simulink model "AcasXuClosedLoop_all_2017b"  (Simulink 9.0)
settings: Solver=VariableStepAuto  StartTime=0.0  StopTime=20  RelTol=1e-3
libraries: neural x14040, simulink x2
(part 23 of 534)

== system AcasXuClosedLoop_all_2017b/networks/Networks_m_5/AcasXu_3_5/Process Output 1 ==
  a : Inport  PortDimensions=5
      mask Display: e = 0.06;c1 = [120 230 180]/255;c2 = ([120 230 180]+0)/(256+128);c3 = ([120 230 180]+128)/(256+128);patch([0 1 1 0],[0 0 1 1],c1);patch([0 1 1 1-e 1-e 0+e],[0 0 1 1-e 0+e 0+e],c2);patch([0 0 1 1-e 0+e 0+e],[0 1 1 1-e 1-e 0+e],c3);plot([0 1 1 0 0],[0 0 1 1 0]);
  y : Outport  PortDimensions=5
      mask Display: e = 0.06;c1 = [120 230 180]/255;c2 = ([120 230 180]+0)/(256+128);c3 = ([120 230 180]+128)/(256+128);patch([0 1 1 0],[0 0 1 1],c1);patch([0 1 1 1-e 1-e 0+e],[0 0 1 1-e 0+e 0+e],c2);patch([0 0 1 1-e 0+e 0+e],[0 1 1 1-e 1-e 0+e],c3);plot([0 1 1 0 0],[0 0 1 1 0]);
  wires:
  a/1 -> y/1

== system AcasXuClosedLoop_all_2017b/networks/Networks_m_5/AcasXu_4_5/Layer 1 ==
  "p{1}" : Inport  PortDimensions=5
      mask Display: e = 0.06;c1 = [120 230 180]/255;c2 = ([120 230 180]+0)/(256+128);c3 = ([120 230 180]+128)/(256+128);patch([0 1 1 0],[0 0 1 1],c1);patch([0 1 1 1-e 1-e 0+e],[0 0 1 1-e 0+e 0+e],c2);patch([0 0 1 1-e 0+e 0+e],[0 1 1 1-e 1-e 0+e],c3);plot([0 1 1 0 0],[0 0 1 1 0]);
  Delays 1 : Subsystem
      mask Display: e = 0.06;c1 = [120 150 230]/255;c2 = ([120 150 230]+0)/383;c3 = ([120 150 230]+128)/383;patch([0 1 1 0],[0 0 1 1],c1);patch([0 1 1 1-e 1-e 0+e],[0 0 1 1-e 0+e 0+e],c2);patch([0 0 1 1-e 0+e 0+e],[0 1 1 1-e 1-e 0+e],c3);plot([0 1 1 0 0],[0 0 1 1 0]);disp('0');
  "IW{1,1}" : Subsystem
      mask Display: e = 0.06;c1 = [120 150 230]/255;c2 = ([120 150 230]+0)/383;c3 = ([120 150 230]+128)/383;patch([0 1 1 0],[0 0 1 1],c1);patch([0 1 1 1-e 1-e 0+e],[0 0 1 1-e 0+e 0+e],c2);patch([0 0 1 1-e 0+e 0+e],[0 1 1 1-e 1-e 0+e],c3);plot([0 1 1 0 0],[0 0 1 1 0]);disp('W');
  "b{1}" : Constant  Value=[-0.55702700000000004987299462300143204629421234130859375;-0.317043000000000019245049998062313534319400787353515625;0.1381040000000000045332626541494391858577728271484375;0.1899780000000000079740658520677243359386920928955078125;0.18814900000000001067945731847430579364299774169921875;0.05523619999999999918838256007802556268870830535888671875;-0.06766999999999999404476369591066031716763973236083984375;-0.260805000000000009041656312547274865210056304931640625;-0.267183000000000003826272632068139500916004180908203125;-0.34851799999999999446487208842881955206394195556640625;-0.61407800000000001272582039746339432895183563232421875;0.2179210000000000035935698861067066900432109832763671875;-0.156318000000000012494894008341361768543720245361328125;-0.191004000000000007108980071279802359640598297119140625;-0.56346700000000005115197154736961238086223602294921875;0.198722000000000009745093620949774049222469329833984375;-0.64252600000000004154543375989305786788463592529296875;-0.057572900000000003239986057224086835049092769622802734375;0.05113020000000000064854788206503144465386867523193359375;-0.187771999999999994468424802107620052993297576904296875;-0.024837800000000000155697676973431953229010105133056640625;-0.02066489999999999993551824672977090813219547271728515625;0.25938600000000000544986278327996842563152313232421875;-0.1834780000000000022009061240169103257358074188232421875;-0.1499230000000000007087663789206999354064464569091796875;0.045113599999999996759125764356213039718568325042724609375;-0.3716880000000000183746351467561908066272735595703125;-0.0188616999999999986059595613596684415824711322784423828125;0.036677700000000000579714054538271739147603511810302734375;-0.552902000000000004575895218295045197010040283203125;0.18997900000000000897415475265006534755229949951171875;-0.3588970000000000215578666029614396393299102783203125;0.256363999999999980783371711368090473115444183349609375;0.138195000000000012274625760255730710923671722412109375;-0.47843400000000002592059900052845478057861328125;0.01338660000000000023512303215511565213091671466827392578125;-0.13313099999999999933919525574310682713985443115234375;-0.34949200000000002486189032424590550363063812255859375;-0.01373609999999999935205163836826613987796008586883544921875;-0.65224700000000002120259523508138954639434814453125;0.1802229999999999943138817570797982625663280487060546875;-0.408499000000000000998312543742940761148929595947265625;-0.42954199999999997938715523559949360787868499755859375;-0.02018129999999999923954163705275277607142925262451171875;0.07101649999999999629540781143077765591442584991455078125;0.06858029999999999681836726495021139271557331085205078125;-0.303010999999999974807707303625647909939289093017578125;-0.0773268000000000010896172852881136350333690643310546875;0.029496399999999999008704065772690228186547756195068359375;-0.2007400000000000017674750552032492123544216156005859375]
      mask Display: e = 0.06;c1 = [120 150 230]/255;c2 = ([120 150 230]+0)/383;c3 = ([120 150 230]+128)/383;patch([0 1 1 0],[0 0 1 1],c1);patch([0 1 1 1-e 1-e 0+e],[0 0 1 1-e 0+e 0+e],c2);patch([0 0 1 1-e 0+e 0+e],[0 1 1 1-e 1-e 0+e],c3);plot([0 1 1 0 0],[0 0 1 1 0]);disp('b');
  netsum : Sum  Inputs=2  SaturateOnIntegerOverflow=on  InputSameDT=on  OutDataTypeStr=Inherit: Same as first input
      mask Display: e = 0.06;c1 = [120 150 230]/255;c2 = ([120 150 230]+0)/383;c3 = ([120 150 230]+128)/383;patch([0 1 1 0],[0 0 1 1],c1);patch([0 1 1 1-e 1-e 0+e],[0 0 1 1-e 0+e 0+e],c2);patch([0 0 1 1-e 0+e 0+e],[0 1 1 1-e 1-e 0+e],c3);plot([0 1 1 0 0],[0 0 1 1 0]);disp('+');
  poslin : neural/Transfer Functions/poslin
      settings: SourceProductName=Deep Learning Toolbox  SourceProductBaseCode=NN
  "a{1}" : Outport  InitialOutput=[0;0;0;0;0;0;0;0;0;0;0;0;0;0;0;0;0;0;0;0;0;0;0;0;0;0;0;0;0;0;0;0;0;0;0;0;0;0;0;0;0;0;0;0;0;0;0;0;0;0]
      mask Display: e = 0.06;c1 = [120 230 180]/255;c2 = ([120 230 180]+0)/(256+128);c3 = ([120 230 180]+128)/(256+128);patch([0 1 1 0],[0 0 1 1],c1);patch([0 1 1 1-e 1-e 0+e],[0 0 1 1-e 0+e 0+e],c2);patch([0 0 1 1-e 0+e 0+e],[0 1 1 1-e 1-e 0+e],c3);plot([0 1 1 0 0],[0 0 1 1 0]);
  wires:
  poslin/1 -> "a{1}"/1
  netsum/1 -> poslin/1
  "b{1}"/1 -> netsum/2
  "IW{1,1}"/1("iz{1,1}") -> netsum/1
  Delays 1/1("pd{1,1}") -> "IW{1,1}"/1("pd{1,1}")
  "p{1}"/1 -> Delays 1/1("p{1}")

== system AcasXuClosedLoop_all_2017b/networks/Networks_m_5/AcasXu_4_5/Layer 2 ==
  "a{1} " : Inport  PortDimensions=50
      mask Display: e = 0.06;c1 = [120 230 180]/255;c2 = ([120 230 180]+0)/(256+128);c3 = ([120 230 180]+128)/(256+128);patch([0 1 1 0],[0 0 1 1],c1);patch([0 1 1 1-e 1-e 0+e],[0 0 1 1-e 0+e 0+e],c2);patch([0 0 1 1-e 0+e 0+e],[0 1 1 1-e 1-e 0+e],c3);plot([0 1 1 0 0],[0 0 1 1 0]);
  Delays 1 : Subsystem
      mask Display: e = 0.06;c1 = [120 150 230]/255;c2 = ([120 150 230]+0)/383;c3 = ([120 150 230]+128)/383;patch([0 1 1 0],[0 0 1 1],c1);patch([0 1 1 1-e 1-e 0+e],[0 0 1 1-e 0+e 0+e],c2);patch([0 0 1 1-e 0+e 0+e],[0 1 1 1-e 1-e 0+e],c3);plot([0 1 1 0 0],[0 0 1 1 0]);disp('0');
  "LW{2,1}" : Subsystem
      mask Display: e = 0.06;c1 = [120 150 230]/255;c2 = ([120 150 230]+0)/383;c3 = ([120 150 230]+128)/383;patch([0 1 1 0],[0 0 1 1],c1);patch([0 1 1 1-e 1-e 0+e],[0 0 1 1-e 0+e 0+e],c2);patch([0 0 1 1-e 0+e 0+e],[0 1 1 1-e 1-e 0+e],c3);plot([0 1 1 0 0],[0 0 1 1 0]);disp('W');
  "b{2}" : Constant  Value=[-0.72558800000000001073630073733511380851268768310546875;-0.0030770799999999998326771777357180326362140476703643798828125;-0.0214728999999999996262101120692022959701716899871826171875;-1.022629999999999927950966593925841152667999267578125;0.013211699999999999832756003570466418750584125518798828125;0.254539000000000015244694395732949487864971160888671875;-0.0161836999999999987809307100405931123532354831695556640625;1.004890000000000060964566728216595947742462158203125;0.366732999999999975671016727574169635772705078125;0.5765860000000000429309920946252532303333282470703125;-1.1223099999999999187849653026205487549304962158203125;-0.1011060000000000014264145420384011231362819671630859375;0.2349920000000000064321881154683069325983524322509765625;0.4978920000000000012363443602225743234157562255859375;0.380083000000000004181543999948189593851566314697265625;-0.1062639999999999973478992387754260562360286712646484375;-0.00875836999999999969712671799015879514627158641815185546875;-0.2173940000000000039026559761623502708971500396728515625;-0.03386909999999999920650139983990811742842197418212890625;0.1742410000000000069864114493611850775778293609619140625;0.9405099999999999571542730336659587919712066650390625;-0.446786999999999989707788472514948807656764984130859375;-0.432205000000000005844214001626824028789997100830078125;-0.67214099999999998846789139861357398331165313720703125;-0.847107999999999972118303048773668706417083740234375;-1.2810200000000000475353090223507024347782135009765625;-0.0116062000000000005439648731453416985459625720977783203125;-0.0695950999999999930789584823287441395223140716552734375;0.76355300000000003723954478118685074150562286376953125;-0.0096115499999999999547473095162786194123327732086181640625;-0.455886999999999986687981845534523017704486846923828125;-0.88311499999999998333777284642565064132213592529296875;-0.1558090000000000030500046932502300478518009185791015625;-0.2641649999999999831601371624856255948543548583984375;1.152320000000000010942358130705542862415313720703125;-0.00141853000000000009465928041407778437132947146892547607421875;-0.1892749999999999988009591334048309363424777984619140625;0.00461054999999999971793673836373272933997213840484619140625;-0.033554899999999998616573293475084938108921051025390625;-0.0259916000000000001202149491064119501970708370208740234375;-0.62039299999999997226041159592568874359130859375;-0.5875559999999999671871364625985734164714813232421875;0.2564100000000000267874611381557770073413848876953125;0.323012000000000021326940213839407078921794891357421875;-0.339326999999999989743315609302953816950321197509765625;0.085024299999999997101696180834551341831684112548828125;-0.09253550000000000663735733041903586126863956451416015625;0.110709000000000001850963826655060984194278717041015625;-0.006517229999999999724702437475798433297313749790191650390625;0.7578899999999999526067995248013176023960113525390625]
      mask Display: e = 0.06;c1 = [120 150 230]/255;c2 = ([120 150 230]+0)/383;c3 = ([120 150 230]+128)/383;patch([0 1 1 0],[0 0 1 1],c1);patch([0 1 1 1-e 1-e 0+e],[0 0 1 1-e 0+e 0+e],c2);patch([0 0 1 1-e 0+e 0+e],[0 1 1 1-e 1-e 0+e],c3);plot([0 1 1 0 0],[0 0 1 1 0]);disp('b');
  netsum : Sum  Inputs=2  SaturateOnIntegerOverflow=on  InputSameDT=on  OutDataTypeStr=Inherit: Same as first input
      mask Display: e = 0.06;c1 = [120 150 230]/255;c2 = ([120 150 230]+0)/383;c3 = ([120 150 230]+128)/383;patch([0 1 1 0],[0 0 1 1],c1);patch([0 1 1 1-e 1-e 0+e],[0 0 1 1-e 0+e 0+e],c2);patch([0 0 1 1-e 0+e 0+e],[0 1 1 1-e 1-e 0+e],c3);plot([0 1 1 0 0],[0 0 1 1 0]);disp('+');
  poslin : neural/Transfer Functions/poslin
      settings: SourceProductName=Deep Learning Toolbox  SourceProductBaseCode=NN
  "a{2}" : Outport  InitialOutput=[0;0;0;0;0;0;0;0;0;0;0;0;0;0;0;0;0;0;0;0;0;0;0;0;0;0;0;0;0;0;0;0;0;0;0;0;0;0;0;0;0;0;0;0;0;0;0;0;0;0]
      mask Display: e = 0.06;c1 = [120 230 180]/255;c2 = ([120 230 180]+0)/(256+128);c3 = ([120 230 180]+128)/(256+128);patch([0 1 1 0],[0 0 1 1],c1);patch([0 1 1 1-e 1-e 0+e],[0 0 1 1-e 0+e 0+e],c2);patch([0 0 1 1-e 0+e 0+e],[0 1 1 1-e 1-e 0+e],c3);plot([0 1 1 0 0],[0 0 1 1 0]);
  wires:
  poslin/1 -> "a{2}"/1
  netsum/1 -> poslin/1
  "b{2}"/1 -> netsum/2
  "LW{2,1}"/1("lz{2,1}") -> netsum/1
  Delays 1/1("pd{2,1}") -> "LW{2,1}"/1("ad{2,1}")
  "a{1} "/1 -> Delays 1/1("p{2}")

== system AcasXuClosedLoop_all_2017b/networks/Networks_m_5/AcasXu_4_5/Layer 3 ==
  "a{2} " : Inport  PortDimensions=50
      mask Display: e = 0.06;c1 = [120 230 180]/255;c2 = ([120 230 180]+0)/(256+128);c3 = ([120 230 180]+128)/(256+128);patch([0 1 1 0],[0 0 1 1],c1);patch([0 1 1 1-e 1-e 0+e],[0 0 1 1-e 0+e 0+e],c2);patch([0 0 1 1-e 0+e 0+e],[0 1 1 1-e 1-e 0+e],c3);plot([0 1 1 0 0],[0 0 1 1 0]);
  Delays 1 : Subsystem
      mask Display: e = 0.06;c1 = [120 150 230]/255;c2 = ([120 150 230]+0)/383;c3 = ([120 150 230]+128)/383;patch([0 1 1 0],[0 0 1 1],c1);patch([0 1 1 1-e 1-e 0+e],[0 0 1 1-e 0+e 0+e],c2);patch([0 0 1 1-e 0+e 0+e],[0 1 1 1-e 1-e 0+e],c3);plot([0 1 1 0 0],[0 0 1 1 0]);disp('0');
  "LW{3,2}" : Subsystem
      mask Display: e = 0.06;c1 = [120 150 230]/255;c2 = ([120 150 230]+0)/383;c3 = ([120 150 230]+128)/383;patch([0 1 1 0],[0 0 1 1],c1);patch([0 1 1 1-e 1-e 0+e],[0 0 1 1-e 0+e 0+e],c2);patch([0 0 1 1-e 0+e 0+e],[0 1 1 1-e 1-e 0+e],c3);plot([0 1 1 0 0],[0 0 1 1 0]);disp('W');
  "b{3}" : Constant  Value=[1.2183299999999999130295691429637372493743896484375;0.3758529999999999926529881122405640780925750732421875;-0.49951800000000001755751100063207559287548065185546875;0.2297079999999999955218044078719685785472393035888671875;-0.2305609999999999881747925201125326566398143768310546875;0.6040140000000000508606490257079713046550750732421875;-0.1264039999999999885904600205321912653744220733642578125;-1.15477000000000007418066161335445940494537353515625;0.9251979999999999648707671440206468105316162109375;0.391276000000000012679635119638987816870212554931640625;-0.392373000000000027309710048939450643956661224365234375;-0.0485302999999999984392928809029399417340755462646484375;0.661183999999999993946175891323946416378021240234375;-0.258817000000000019266366280135116539895534515380859375;-0.2621430000000000148929757415316998958587646484375;-0.421345000000000025064395003937534056603908538818359375;-2.23233000000000014750867194379679858684539794921875;-0.402540999999999982161824618742684833705425262451171875;-1.2703599999999999337063627535826526582241058349609375;0.62634800000000001585220843480783514678478240966796875;-1.4001799999999999801758576722932048141956329345703125;-0.9811549999999999993605115378159098327159881591796875;-1.5831999999999999406696815640316344797611236572265625;0.06447740000000000415436574030536576174199581146240234375;0.269380000000000008331113576787174679338932037353515625;-0.363032999999999994589217067186837084591388702392578125;-0.00779845000000000017348344982792696100659668445587158203125;-0.55507899999999998907895815136726014316082000732421875;0.85643199999999997107380522720632143318653106689453125;0.2440710000000000101660901918876334093511104583740234375;-0.340949000000000002064126647383091039955615997314453125;1.81261999999999989796606314484961330890655517578125;0.86188100000000000822097945274435915052890777587890625;0.70267800000000002480504690538509748876094818115234375;-2.120080000000000186588522410602308809757232666015625;-0.47471900000000000208189021577709354460239410400390625;0.73649100000000000676436684443615376949310302734375;-0.19158500000000000529354338141274638473987579345703125;-0.99656299999999997663024942085030488669872283935546875;-1.2982299999999999950972551232553087174892425537109375;-0.3749730000000000007531752999057061970233917236328125;0.355833000000000010398792937849066220223903656005859375;0.46674300000000001897859647215227596461772918701171875;0.195896999999999987807086654356680810451507568359375;-0.2098629999999999939941375259877531789243221282958984375;1.44169999999999998152588887023739516735076904296875;-1.926909999999999900666125540737994015216827392578125;-1.3318600000000000438404867963981814682483673095703125;-0.60810799999999998188826566547504626214504241943359375;-0.055248899999999996623945008877853979356586933135986328125]
      mask Display: e = 0.06;c1 = [120 150 230]/255;c2 = ([120 150 230]+0)/383;c3 = ([120 150 230]+128)/383;patch([0 1 1 0],[0 0 1 1],c1);patch([0 1 1 1-e 1-e 0+e],[0 0 1 1-e 0+e 0+e],c2);patch([0 0 1 1-e 0+e 0+e],[0 1 1 1-e 1-e 0+e],c3);plot([0 1 1 0 0],[0 0 1 1 0]);disp('b');
  netsum : Sum  Inputs=2  SaturateOnIntegerOverflow=on  InputSameDT=on  OutDataTypeStr=Inherit: Same as first input
      mask Display: e = 0.06;c1 = [120 150 230]/255;c2 = ([120 150 230]+0)/383;c3 = ([120 150 230]+128)/383;patch([0 1 1 0],[0 0 1 1],c1);patch([0 1 1 1-e 1-e 0+e],[0 0 1 1-e 0+e 0+e],c2);patch([0 0 1 1-e 0+e 0+e],[0 1 1 1-e 1-e 0+e],c3);plot([0 1 1 0 0],[0 0 1 1 0]);disp('+');
  poslin : neural/Transfer Functions/poslin
      settings: SourceProductName=Deep Learning Toolbox  SourceProductBaseCode=NN
  "a{3}" : Outport  InitialOutput=[0;0;0;0;0;0;0;0;0;0;0;0;0;0;0;0;0;0;0;0;0;0;0;0;0;0;0;0;0;0;0;0;0;0;0;0;0;0;0;0;0;0;0;0;0;0;0;0;0;0]
      mask Display: e = 0.06;c1 = [120 230 180]/255;c2 = ([120 230 180]+0)/(256+128);c3 = ([120 230 180]+128)/(256+128);patch([0 1 1 0],[0 0 1 1],c1);patch([0 1 1 1-e 1-e 0+e],[0 0 1 1-e 0+e 0+e],c2);patch([0 0 1 1-e 0+e 0+e],[0 1 1 1-e 1-e 0+e],c3);plot([0 1 1 0 0],[0 0 1 1 0]);
  wires:
  poslin/1 -> "a{3}"/1
  netsum/1 -> poslin/1
  "b{3}"/1 -> netsum/2
  "LW{3,2}"/1("lz{3,2}") -> netsum/1
  Delays 1/1("pd{3,2}") -> "LW{3,2}"/1("ad{3,2}")
  "a{2} "/1 -> Delays 1/1("p{3}")

== system AcasXuClosedLoop_all_2017b/networks/Networks_m_5/AcasXu_4_5/Layer 4 ==
  "a{3} " : Inport  PortDimensions=50
      mask Display: e = 0.06;c1 = [120 230 180]/255;c2 = ([120 230 180]+0)/(256+128);c3 = ([120 230 180]+128)/(256+128);patch([0 1 1 0],[0 0 1 1],c1);patch([0 1 1 1-e 1-e 0+e],[0 0 1 1-e 0+e 0+e],c2);patch([0 0 1 1-e 0+e 0+e],[0 1 1 1-e 1-e 0+e],c3);plot([0 1 1 0 0],[0 0 1 1 0]);
  Delays 1 : Subsystem
      mask Display: e = 0.06;c1 = [120 150 230]/255;c2 = ([120 150 230]+0)/383;c3 = ([120 150 230]+128)/383;patch([0 1 1 0],[0 0 1 1],c1);patch([0 1 1 1-e 1-e 0+e],[0 0 1 1-e 0+e 0+e],c2);patch([0 0 1 1-e 0+e 0+e],[0 1 1 1-e 1-e 0+e],c3);plot([0 1 1 0 0],[0 0 1 1 0]);disp('0');
  "LW{4,3}" : Subsystem
      mask Display: e = 0.06;c1 = [120 150 230]/255;c2 = ([120 150 230]+0)/383;c3 = ([120 150 230]+128)/383;patch([0 1 1 0],[0 0 1 1],c1);patch([0 1 1 1-e 1-e 0+e],[0 0 1 1-e 0+e 0+e],c2);patch([0 0 1 1-e 0+e 0+e],[0 1 1 1-e 1-e 0+e],c3);plot([0 1 1 0 0],[0 0 1 1 0]);disp('W');
  "b{4}" : Constant  Value=[-1.13694000000000006167510946397669613361358642578125;-1.44306000000000000937916411203332245349884033203125;-0.460143000000000024218849148383014835417270660400390625;-0.0907355999999999995875299418912618421018123626708984375;0.2415279999999999926973970332255703397095203399658203125;-0.192749000000000003662847802843316458165645599365234375;-0.6306410000000000071196382123162038624286651611328125;0.83967000000000002746247673712787218391895294189453125;-1.3998500000000000387245790989254601299762725830078125;0.0822589999999999987867482786896289326250553131103515625;0.64448600000000000331823457599966786801815032958984375;0.346046000000000020246915255484054796397686004638671875;-1.373180000000000067217342802905477583408355712890625;1.917710000000000025721647034515626728534698486328125;1.3785000000000000586197757002082653343677520751953125;1.09443000000000001392663762089796364307403564453125;1.95282000000000000028421709430404007434844970703125;0.1447380000000000055404569820893811993300914764404296875;-2.013619999999999965467623042059130966663360595703125;0.333592999999999972882136489715776406228542327880859375;-0.01296309999999999997000177387462827027775347232818603515625;1.175580000000000069348971010185778141021728515625;0.07237180000000000001936228954946273006498813629150390625;-1.059649999999999980815346134477294981479644775390625;-0.9808179999999999676418838134850375354290008544921875;-3.806229999999999780158077555825002491474151611328125;-2.06843999999999983430143402074463665485382080078125;-3.407760000000000122355459097889252007007598876953125;-1.9770700000000001050892706189188174903392791748046875;0.5420409999999999950404117043945007026195526123046875;-2.08537000000000016797230273368768393993377685546875;-2.5549400000000002108890839735977351665496826171875;-1.272710000000000007958078640513122081756591796875;1.8852100000000000523669996255193836987018585205078125;0.417991999999999974679809611188829876482486724853515625;-1.0045699999999999629807234668987803161144256591796875;4.056779999999999830606611794792115688323974609375;-1.4069599999999999884181534071103669703006744384765625;-1.2732200000000000184030568561865948140621185302734375;0.00521946000000000041862957544935852638445794582366943359375;-0.06468649999999999400390748860445455648005008697509765625;-0.11416099999999999858868449109650100581347942352294921875;-0.78644800000000003592504072003066539764404296875;1.2535000000000000586197757002082653343677520751953125;-1.5074199999999999821653773324214853346347808837890625;-0.2420319999999999971418418454049970023334026336669921875;1.3262300000000000199662508748588152229785919189453125;0.19241099999999999869970679355901665985584259033203125;-0.0200379000000000008496758852061248035170137882232666015625;-1.84077000000000001733724275254644453525543212890625]
      mask Display: e = 0.06;c1 = [120 150 230]/255;c2 = ([120 150 230]+0)/383;c3 = ([120 150 230]+128)/383;patch([0 1 1 0],[0 0 1 1],c1);patch([0 1 1 1-e 1-e 0+e],[0 0 1 1-e 0+e 0+e],c2);patch([0 0 1 1-e 0+e 0+e],[0 1 1 1-e 1-e 0+e],c3);plot([0 1 1 0 0],[0 0 1 1 0]);disp('b');
  netsum : Sum  Inputs=2  SaturateOnIntegerOverflow=on  InputSameDT=on  OutDataTypeStr=Inherit: Same as first input
      mask Display: e = 0.06;c1 = [120 150 230]/255;c2 = ([120 150 230]+0)/383;c3 = ([120 150 230]+128)/383;patch([0 1 1 0],[0 0 1 1],c1);patch([0 1 1 1-e 1-e 0+e],[0 0 1 1-e 0+e 0+e],c2);patch([0 0 1 1-e 0+e 0+e],[0 1 1 1-e 1-e 0+e],c3);plot([0 1 1 0 0],[0 0 1 1 0]);disp('+');
  poslin : neural/Transfer Functions/poslin
      settings: SourceProductName=Deep Learning Toolbox  SourceProductBaseCode=NN
  "a{4}" : Outport  InitialOutput=[0;0;0;0;0;0;0;0;0;0;0;0;0;0;0;0;0;0;0;0;0;0;0;0;0;0;0;0;0;0;0;0;0;0;0;0;0;0;0;0;0;0;0;0;0;0;0;0;0;0]
      mask Display: e = 0.06;c1 = [120 230 180]/255;c2 = ([120 230 180]+0)/(256+128);c3 = ([120 230 180]+128)/(256+128);patch([0 1 1 0],[0 0 1 1],c1);patch([0 1 1 1-e 1-e 0+e],[0 0 1 1-e 0+e 0+e],c2);patch([0 0 1 1-e 0+e 0+e],[0 1 1 1-e 1-e 0+e],c3);plot([0 1 1 0 0],[0 0 1 1 0]);
  wires:
  poslin/1 -> "a{4}"/1
  netsum/1 -> poslin/1
  "b{4}"/1 -> netsum/2
  "LW{4,3}"/1("lz{4,3}") -> netsum/1
  Delays 1/1("pd{4,3}") -> "LW{4,3}"/1("ad{4,3}")
  "a{3} "/1 -> Delays 1/1("p{4}")

== system AcasXuClosedLoop_all_2017b/networks/Networks_m_5/AcasXu_4_5/Layer 5 ==
  "a{4} " : Inport  PortDimensions=50
      mask Display: e = 0.06;c1 = [120 230 180]/255;c2 = ([120 230 180]+0)/(256+128);c3 = ([120 230 180]+128)/(256+128);patch([0 1 1 0],[0 0 1 1],c1);patch([0 1 1 1-e 1-e 0+e],[0 0 1 1-e 0+e 0+e],c2);patch([0 0 1 1-e 0+e 0+e],[0 1 1 1-e 1-e 0+e],c3);plot([0 1 1 0 0],[0 0 1 1 0]);
  Delays 1 : Subsystem
      mask Display: e = 0.06;c1 = [120 150 230]/255;c2 = ([120 150 230]+0)/383;c3 = ([120 150 230]+128)/383;patch([0 1 1 0],[0 0 1 1],c1);patch([0 1 1 1-e 1-e 0+e],[0 0 1 1-e 0+e 0+e],c2);patch([0 0 1 1-e 0+e 0+e],[0 1 1 1-e 1-e 0+e],c3);plot([0 1 1 0 0],[0 0 1 1 0]);disp('0');
  "LW{5,4}" : Subsystem
      mask Display: e = 0.06;c1 = [120 150 230]/255;c2 = ([120 150 230]+0)/383;c3 = ([120 150 230]+128)/383;patch([0 1 1 0],[0 0 1 1],c1);patch([0 1 1 1-e 1-e 0+e],[0 0 1 1-e 0+e 0+e],c2);patch([0 0 1 1-e 0+e 0+e],[0 1 1 1-e 1-e 0+e],c3);plot([0 1 1 0 0],[0 0 1 1 0]);disp('W');
  "b{5}" : Constant  Value=[-0.005314890000000000065683014582873511244542896747589111328125;1.0904199999999999448618837050162255764007568359375;2.140670000000000072759576141834259033203125;1.008620000000000072049033406074158847332000732421875;2.096159999999999801190142534323967993259429931640625;2.171110000000000095354835139005444943904876708984375;0.60804800000000003290523409305023960769176483154296875;-0.4575299999999999922550841802149079740047454833984375;2.1910199999999999675992512493394315242767333984375;-1.6480799999999999894129132371745072305202484130859375;-0.499765999999999988023802188763511367142200469970703125;0.85345199999999998841104797975276596844196319580078125;2.008459999999999912034809312899596989154815673828125;-0.0260502999999999984570564492969424463808536529541015625;2.183240000000000069491079557337798178195953369140625;0.2656549999999999744915157862124033272266387939453125;0.9520910000000000206199501917581073939800262451171875;0.5391049999999999453592636200482957065105438232421875;3.45282000000000000028421709430404007434844970703125;0.6617189999999999461266497746692039072513580322265625;-0.81202600000000002555822220529080368578433990478515625;-0.66264599999999995727506529874517582356929779052734375;-0.038156900000000000539035482916006003506481647491455078125;3.081989999999999785273985253297723829746246337890625;0.259077000000000001733724275254644453525543212890625;0.5673280000000000544702061233692802488803863525390625;0.11513299999999999922994931011999142356216907501220703125;4.59682000000000012818190953112207353115081787109375;0;-1.0043699999999999850075482754618860781192779541015625;-2.511760000000000214726014746702276170253753662109375;0.59331999999999995853983136839815415441989898681640625;1.174630000000000062954086388344876468181610107421875;0.49534000000000000252242671194835565984249114990234375;-1.8436500000000000110134124042815528810024261474609375;2.802049999999999929656269159750081598758697509765625;-0.397106000000000014527046232615248300135135650634765625;-0.168563999999999991619148431709618307650089263916015625;1.7117500000000001048050535246147774159908294677734375;1.1961500000000000465405491922865621745586395263671875;1.292710000000000025721647034515626728534698486328125;2.4907599999999998630073605454526841640472412109375;-0.335679999999999978399500832892954349517822265625;1.2010099999999999109689952092594467103481292724609375;0.98422500000000001652011860642232932150363922119140625;0.92655200000000004223466021358035504817962646484375;3.378190000000000026147972675971686840057373046875;1.092610000000000081143980423803441226482391357421875;-1.2016899999999999248956328301574103534221649169921875;0.1211689999999999989288568258416489697992801666259765625]
      mask Display: e = 0.06;c1 = [120 150 230]/255;c2 = ([120 150 230]+0)/383;c3 = ([120 150 230]+128)/383;patch([0 1 1 0],[0 0 1 1],c1);patch([0 1 1 1-e 1-e 0+e],[0 0 1 1-e 0+e 0+e],c2);patch([0 0 1 1-e 0+e 0+e],[0 1 1 1-e 1-e 0+e],c3);plot([0 1 1 0 0],[0 0 1 1 0]);disp('b');
  netsum : Sum  Inputs=2  SaturateOnIntegerOverflow=on  InputSameDT=on  OutDataTypeStr=Inherit: Same as first input
      mask Display: e = 0.06;c1 = [120 150 230]/255;c2 = ([120 150 230]+0)/383;c3 = ([120 150 230]+128)/383;patch([0 1 1 0],[0 0 1 1],c1);patch([0 1 1 1-e 1-e 0+e],[0 0 1 1-e 0+e 0+e],c2);patch([0 0 1 1-e 0+e 0+e],[0 1 1 1-e 1-e 0+e],c3);plot([0 1 1 0 0],[0 0 1 1 0]);disp('+');
  poslin : neural/Transfer Functions/poslin
      settings: SourceProductName=Deep Learning Toolbox  SourceProductBaseCode=NN
  "a{5}" : Outport  InitialOutput=[0;0;0;0;0;0;0;0;0;0;0;0;0;0;0;0;0;0;0;0;0;0;0;0;0;0;0;0;0;0;0;0;0;0;0;0;0;0;0;0;0;0;0;0;0;0;0;0;0;0]
      mask Display: e = 0.06;c1 = [120 230 180]/255;c2 = ([120 230 180]+0)/(256+128);c3 = ([120 230 180]+128)/(256+128);patch([0 1 1 0],[0 0 1 1],c1);patch([0 1 1 1-e 1-e 0+e],[0 0 1 1-e 0+e 0+e],c2);patch([0 0 1 1-e 0+e 0+e],[0 1 1 1-e 1-e 0+e],c3);plot([0 1 1 0 0],[0 0 1 1 0]);
  wires:
  poslin/1 -> "a{5}"/1
  netsum/1 -> poslin/1
  "b{5}"/1 -> netsum/2
  "LW{5,4}"/1("lz{5,4}") -> netsum/1
  Delays 1/1("pd{5,4}") -> "LW{5,4}"/1("ad{5,4}")
  "a{4} "/1 -> Delays 1/1("p{5}")

== system AcasXuClosedLoop_all_2017b/networks/Networks_m_5/AcasXu_4_5/Layer 6 ==
  "a{5} " : Inport  PortDimensions=50
      mask Display: e = 0.06;c1 = [120 230 180]/255;c2 = ([120 230 180]+0)/(256+128);c3 = ([120 230 180]+128)/(256+128);patch([0 1 1 0],[0 0 1 1],c1);patch([0 1 1 1-e 1-e 0+e],[0 0 1 1-e 0+e 0+e],c2);patch([0 0 1 1-e 0+e 0+e],[0 1 1 1-e 1-e 0+e],c3);plot([0 1 1 0 0],[0 0 1 1 0]);
  Delays 1 : Subsystem
      mask Display: e = 0.06;c1 = [120 150 230]/255;c2 = ([120 150 230]+0)/383;c3 = ([120 150 230]+128)/383;patch([0 1 1 0],[0 0 1 1],c1);patch([0 1 1 1-e 1-e 0+e],[0 0 1 1-e 0+e 0+e],c2);patch([0 0 1 1-e 0+e 0+e],[0 1 1 1-e 1-e 0+e],c3);plot([0 1 1 0 0],[0 0 1 1 0]);disp('0');
  "LW{6,5}" : Subsystem
      mask Display: e = 0.06;c1 = [120 150 230]/255;c2 = ([120 150 230]+0)/383;c3 = ([120 150 230]+128)/383;patch([0 1 1 0],[0 0 1 1],c1);patch([0 1 1 1-e 1-e 0+e],[0 0 1 1-e 0+e 0+e],c2);patch([0 0 1 1-e 0+e 0+e],[0 1 1 1-e 1-e 0+e],c3);plot([0 1 1 0 0],[0 0 1 1 0]);disp('W');
  "b{6}" : Constant  Value=[0.2259790000000000131930022462256602011620998382568359375;0.64113699999999995693400478558032773435115814208984375;-1.122030000000000082849282989627681672573089599609375;-0.258402999999999993807620057850726880133152008056640625;0.279403999999999985703880156506784260272979736328125;-3.158129999999999881765688769519329071044921875;3.81989000000000000767386154620908200740814208984375;-0.83881700000000003480948862488730810582637786865234375;0.33712900000000001199396137963049113750457763671875;1.481430000000000024584778657299466431140899658203125;-0.387311000000000016374457345591508783400058746337890625;-4.85249000000000041410430640098638832569122314453125;-1.463179999999999925108795650885440409183502197265625;-0.62209700000000001107736125049996189773082733154296875;-5.7391600000000000392219590139575302600860595703125;-0.53950500000000001232791646543773822486400604248046875;0.355816999999999994397370528531610034406185150146484375;-3.2089400000000001256239556823857128620147705078125;-0.66979200000000005399414249041001312434673309326171875;-0.053271800000000001096278623435864574275910854339599609375;-1.0725899999999999323563315556384623050689697265625;0.2332220000000000126316734849751810543239116668701171875;0.54013299999999997424993125605396926403045654296875;0.034703799999999999925659466271099518053233623504638671875;-1.8084800000000000874678107720683328807353973388671875;-1.0016700000000000603250782660325057804584503173828125;-1.9715800000000001102051783163915388286113739013671875;1.993749999999999911182158029987476766109466552734375;0.66732599999999997475441659844364039599895477294921875;-2.41988000000000003097966327914036810398101806640625;-0.262247999999999981124432224532938562333583831787109375;-2.322389999999999954383156364201568067073822021484375;-6.11990999999999996106225808034650981426239013671875;-2.560119999999999951256768326857127249240875244140625;0.2439320000000000099316110890868003480136394500732421875;-0.2257039999999999879687351267421036027371883392333984375;-0.1239760000000000028652635819526039995253086090087890625;1.8548400000000000442668124378542415797710418701171875;-0.4922050000000000036237679523765109479427337646484375;-0.57257499999999994511057366253226064145565032958984375;-0.53078599999999998004795997985638678073883056640625;-0.59304900000000004833822231375961564481258392333984375;0.0029524999999999998294419878419603264774195849895477294921875;-3.167949999999999821653773324214853346347808837890625;-0.80684900000000003839062401311821304261684417724609375;-3.264060000000000183462134373257867991924285888671875;-0.874765000000000014779288903810083866119384765625;-2.214059999999999917008608463220298290252685546875;0.2967400000000000037658764995285309851169586181640625;-5.6659699999999997288568920339457690715789794921875]
      mask Display: e = 0.06;c1 = [120 150 230]/255;c2 = ([120 150 230]+0)/383;c3 = ([120 150 230]+128)/383;patch([0 1 1 0],[0 0 1 1],c1);patch([0 1 1 1-e 1-e 0+e],[0 0 1 1-e 0+e 0+e],c2);patch([0 0 1 1-e 0+e 0+e],[0 1 1 1-e 1-e 0+e],c3);plot([0 1 1 0 0],[0 0 1 1 0]);disp('b');
  netsum : Sum  Inputs=2  SaturateOnIntegerOverflow=on  InputSameDT=on  OutDataTypeStr=Inherit: Same as first input
      mask Display: e = 0.06;c1 = [120 150 230]/255;c2 = ([120 150 230]+0)/383;c3 = ([120 150 230]+128)/383;patch([0 1 1 0],[0 0 1 1],c1);patch([0 1 1 1-e 1-e 0+e],[0 0 1 1-e 0+e 0+e],c2);patch([0 0 1 1-e 0+e 0+e],[0 1 1 1-e 1-e 0+e],c3);plot([0 1 1 0 0],[0 0 1 1 0]);disp('+');
  poslin : neural/Transfer Functions/poslin
      settings: SourceProductName=Deep Learning Toolbox  SourceProductBaseCode=NN
  "a{6}" : Outport  InitialOutput=[0;0;0;0;0;0;0;0;0;0;0;0;0;0;0;0;0;0;0;0;0;0;0;0;0;0;0;0;0;0;0;0;0;0;0;0;0;0;0;0;0;0;0;0;0;0;0;0;0;0]
      mask Display: e = 0.06;c1 = [120 230 180]/255;c2 = ([120 230 180]+0)/(256+128);c3 = ([120 230 180]+128)/(256+128);patch([0 1 1 0],[0 0 1 1],c1);patch([0 1 1 1-e 1-e 0+e],[0 0 1 1-e 0+e 0+e],c2);patch([0 0 1 1-e 0+e 0+e],[0 1 1 1-e 1-e 0+e],c3);plot([0 1 1 0 0],[0 0 1 1 0]);
  wires:
  poslin/1 -> "a{6}"/1
  netsum/1 -> poslin/1
  "b{6}"/1 -> netsum/2
  "LW{6,5}"/1("lz{6,5}") -> netsum/1
  Delays 1/1("pd{6,5}") -> "LW{6,5}"/1("ad{6,5}")
  "a{5} "/1 -> Delays 1/1("p{6}")

== system AcasXuClosedLoop_all_2017b/networks/Networks_m_5/AcasXu_4_5/Layer 7 ==
  "a{6} " : Inport  PortDimensions=50
      mask Display: e = 0.06;c1 = [120 230 180]/255;c2 = ([120 230 180]+0)/(256+128);c3 = ([120 230 180]+128)/(256+128);patch([0 1 1 0],[0 0 1 1],c1);patch([0 1 1 1-e 1-e 0+e],[0 0 1 1-e 0+e 0+e],c2);patch([0 0 1 1-e 0+e 0+e],[0 1 1 1-e 1-e 0+e],c3);plot([0 1 1 0 0],[0 0 1 1 0]);
  Delays 1 : Subsystem
      mask Display: e = 0.06;c1 = [120 150 230]/255;c2 = ([120 150 230]+0)/383;c3 = ([120 150 230]+128)/383;patch([0 1 1 0],[0 0 1 1],c1);patch([0 1 1 1-e 1-e 0+e],[0 0 1 1-e 0+e 0+e],c2);patch([0 0 1 1-e 0+e 0+e],[0 1 1 1-e 1-e 0+e],c3);plot([0 1 1 0 0],[0 0 1 1 0]);disp('0');
  "LW{7,6}" : Subsystem
      mask Display: e = 0.06;c1 = [120 150 230]/255;c2 = ([120 150 230]+0)/383;c3 = ([120 150 230]+128)/383;patch([0 1 1 0],[0 0 1 1],c1);patch([0 1 1 1-e 1-e 0+e],[0 0 1 1-e 0+e 0+e],c2);patch([0 0 1 1-e 0+e 0+e],[0 1 1 1-e 1-e 0+e],c3);plot([0 1 1 0 0],[0 0 1 1 0]);disp('W');
  "b{7}" : Constant  Value=[-0.0206514999999999997182253963501352700404822826385498046875;-0.0190315999999999992120525149630339001305401325225830078125;0.01813789999999999846824749738516402430832386016845703125;-0.01843229999999999868531830315987463109195232391357421875;0.018363999999999998380406651676821638830006122589111328125]
      mask Display: e = 0.06;c1 = [120 150 230]/255;c2 = ([120 150 230]+0)/383;c3 = ([120 150 230]+128)/383;patch([0 1 1 0],[0 0 1 1],c1);patch([0 1 1 1-e 1-e 0+e],[0 0 1 1-e 0+e 0+e],c2);patch([0 0 1 1-e 0+e 0+e],[0 1 1 1-e 1-e 0+e],c3);plot([0 1 1 0 0],[0 0 1 1 0]);disp('b');
  netsum : Sum  Inputs=2  SaturateOnIntegerOverflow=on  InputSameDT=on  OutDataTypeStr=Inherit: Same as first input
      mask Display: e = 0.06;c1 = [120 150 230]/255;c2 = ([120 150 230]+0)/383;c3 = ([120 150 230]+128)/383;patch([0 1 1 0],[0 0 1 1],c1);patch([0 1 1 1-e 1-e 0+e],[0 0 1 1-e 0+e 0+e],c2);patch([0 0 1 1-e 0+e 0+e],[0 1 1 1-e 1-e 0+e],c3);plot([0 1 1 0 0],[0 0 1 1 0]);disp('+');
  purelin : neural/Transfer Functions/purelin
      settings: SourceProductName=Deep Learning Toolbox
  "a{7}" : Outport  InitialOutput=[0;0;0;0;0]
      mask Display: e = 0.06;c1 = [120 230 180]/255;c2 = ([120 230 180]+0)/(256+128);c3 = ([120 230 180]+128)/(256+128);patch([0 1 1 0],[0 0 1 1],c1);patch([0 1 1 1-e 1-e 0+e],[0 0 1 1-e 0+e 0+e],c2);patch([0 0 1 1-e 0+e 0+e],[0 1 1 1-e 1-e 0+e],c3);plot([0 1 1 0 0],[0 0 1 1 0]);
  wires:
  purelin/1 -> "a{7}"/1
  netsum/1 -> purelin/1
  "b{7}"/1 -> netsum/2
  "LW{7,6}"/1("lz{7,6}") -> netsum/1
  Delays 1/1("pd{7,6}") -> "LW{7,6}"/1("ad{7,6}")
  "a{6} "/1 -> Delays 1/1("p{7}")

== system AcasXuClosedLoop_all_2017b/networks/Networks_m_5/AcasXu_4_5/Process Input 1 ==
  x : Inport  PortDimensions=5
      mask Display: e = 0.06;c1 = [120 230 180]/255;c2 = ([120 230 180]+0)/(256+128);c3 = ([120 230 180]+128)/(256+128);patch([0 1 1 0],[0 0 1 1],c1);patch([0 1 1 1-e 1-e 0+e],[0 0 1 1-e 0+e 0+e],c2);patch([0 0 1 1-e 0+e 0+e],[0 1 1 1-e 1-e 0+e],c3);plot([0 1 1 0 0],[0 0 1 1 0]);
  p : Outport  PortDimensions=5
      mask Display: e = 0.06;c1 = [120 230 180]/255;c2 = ([120 230 180]+0)/(256+128);c3 = ([120 230 180]+128)/(256+128);patch([0 1 1 0],[0 0 1 1],c1);patch([0 1 1 1-e 1-e 0+e],[0 0 1 1-e 0+e 0+e],c2);patch([0 0 1 1-e 0+e 0+e],[0 1 1 1-e 1-e 0+e],c3);plot([0 1 1 0 0],[0 0 1 1 0]);
  wires:
  x/1 -> p/1

== system AcasXuClosedLoop_all_2017b/networks/Networks_m_5/AcasXu_4_5/Process Output 1 ==
  a : Inport  PortDimensions=5
      mask Display: e = 0.06;c1 = [120 230 180]/255;c2 = ([120 230 180]+0)/(256+128);c3 = ([120 230 180]+128)/(256+128);patch([0 1 1 0],[0 0 1 1],c1);patch([0 1 1 1-e 1-e 0+e],[0 0 1 1-e 0+e 0+e],c2);patch([0 0 1 1-e 0+e 0+e],[0 1 1 1-e 1-e 0+e],c3);plot([0 1 1 0 0],[0 0 1 1 0]);
  y : Outport  PortDimensions=5
      mask Display: e = 0.06;c1 = [120 230 180]/255;c2 = ([120 230 180]+0)/(256+128);c3 = ([120 230 180]+128)/(256+128);patch([0 1 1 0],[0 0 1 1],c1);patch([0 1 1 1-e 1-e 0+e],[0 0 1 1-e 0+e 0+e],c2);patch([0 0 1 1-e 0+e 0+e],[0 1 1 1-e 1-e 0+e],c3);plot([0 1 1 0 0],[0 0 1 1 0]);
  wires:
  a/1 -> y/1

== system AcasXuClosedLoop_all_2017b/networks/Networks_m_5/AcasXu_5_5/Layer 1 ==
  "p{1}" : Inport  PortDimensions=5
      mask Display: e = 0.06;c1 = [120 230 180]/255;c2 = ([120 230 180]+0)/(256+128);c3 = ([120 230 180]+128)/(256+128);patch([0 1 1 0],[0 0 1 1],c1);patch([0 1 1 1-e 1-e 0+e],[0 0 1 1-e 0+e 0+e],c2);patch([0 0 1 1-e 0+e 0+e],[0 1 1 1-e 1-e 0+e],c3);plot([0 1 1 0 0],[0 0 1 1 0]);
  Delays 1 : Subsystem
      mask Display: e = 0.06;c1 = [120 150 230]/255;c2 = ([120 150 230]+0)/383;c3 = ([120 150 230]+128)/383;patch([0 1 1 0],[0 0 1 1],c1);patch([0 1 1 1-e 1-e 0+e],[0 0 1 1-e 0+e 0+e],c2);patch([0 0 1 1-e 0+e 0+e],[0 1 1 1-e 1-e 0+e],c3);plot([0 1 1 0 0],[0 0 1 1 0]);disp('0');
  "IW{1,1}" : Subsystem
      mask Display: e = 0.06;c1 = [120 150 230]/255;c2 = ([120 150 230]+0)/383;c3 = ([120 150 230]+128)/383;patch([0 1 1 0],[0 0 1 1],c1);patch([0 1 1 1-e 1-e 0+e],[0 0 1 1-e 0+e 0+e],c2);patch([0 0 1 1-e 0+e 0+e],[0 1 1 1-e 1-e 0+e],c3);plot([0 1 1 0 0],[0 0 1 1 0]);disp('W');
  "b{1}" : Constant  Value=[-0.0753751000000000004330757974457810632884502410888671875;-0.258496000000000003549160965121700428426265716552734375;0.0450709999999999999520383653361932374536991119384765625;-0.160831000000000001737276988933444954454898834228515625;0.132523000000000001907807245515868999063968658447265625;-0.21419799999999999950972551232553087174892425537109375;0.259344999999999992201793475032900460064411163330078125;0.246684999999999987618792829380254261195659637451171875;-0.348399999999999987476684282228234224021434783935546875;-0.10470200000000000339905881219237926416099071502685546875;-0.441989000000000020751400597873725928366184234619140625;-0.382651000000000018896884057539864443242549896240234375;-0.308284999999999975717202005398576147854328155517578125;-0.348181999999999991501908880309201776981353759765625;0.03626870000000000093809404688727227039635181427001953125;0.02015329999999999899262803637611796148121356964111328125;-0.55298800000000003507238943711854517459869384765625;-0.670382000000000033423930290155112743377685546875;-0.6342710000000000292175172944553196430206298828125;-0.302954000000000001069366817318950779736042022705078125;-0.7268769999999999953388396534137427806854248046875;0.2401870000000000116013865181230357848107814788818359375;-0.2027190000000000102975405980032519437372684478759765625;-0.205303999999999986503240734236896969377994537353515625;0.42742000000000002213340621892712078988552093505859375;-0.333015000000000005453415496958768926560878753662109375;0.041898299999999999432276354127679951488971710205078125;0.28865899999999999891997504164464771747589111328125;0.038349500000000001642685987235381617210805416107177734375;0.2934010000000000228936869461904279887676239013671875;-0.088504799999999994586374896243796683847904205322265625;0.22462999999999999634070491083548404276371002197265625;-0.41700999999999999179323140197084285318851470947265625;-0.0162272000000000006514344619290568516589701175689697265625;-0.11031299999999999439381781485280953347682952880859375;-0.5270829999999999682103180020931176841259002685546875;0.06332559999999999578168541347622522152960300445556640625;0.204818000000000000060396132539608515799045562744140625;-0.48429800000000000625988150204648263752460479736328125;0.1990089999999999914592763161635957658290863037109375;-0.07904250000000000164757096854373230598866939544677734375;0.11608000000000000262456723021387006156146526336669921875;-0.52808200000000005136513436809764243662357330322265625;-0.56545100000000003692690597745240665972232818603515625;-0.1539069999999999882600576484037446789443492889404296875;-0.326313999999999992951416061259806156158447265625;-0.0184588000000000009015455049166121170856058597564697265625;-0.1825579999999999980531129040173254907131195068359375;-0.0081059300000000007735412310694300686009228229522705078125;-0.3226620000000000043627323975670151412487030029296875]
      mask Display: e = 0.06;c1 = [120 150 230]/255;c2 = ([120 150 230]+0)/383;c3 = ([120 150 230]+128)/383;patch([0 1 1 0],[0 0 1 1],c1);patch([0 1 1 1-e 1-e 0+e],[0 0 1 1-e 0+e 0+e],c2);patch([0 0 1 1-e 0+e 0+e],[0 1 1 1-e 1-e 0+e],c3);plot([0 1 1 0 0],[0 0 1 1 0]);disp('b');
  netsum : Sum  Inputs=2  SaturateOnIntegerOverflow=on  InputSameDT=on  OutDataTypeStr=Inherit: Same as first input
      mask Display: e = 0.06;c1 = [120 150 230]/255;c2 = ([120 150 230]+0)/383;c3 = ([120 150 230]+128)/383;patch([0 1 1 0],[0 0 1 1],c1);patch([0 1 1 1-e 1-e 0+e],[0 0 1 1-e 0+e 0+e],c2);patch([0 0 1 1-e 0+e 0+e],[0 1 1 1-e 1-e 0+e],c3);plot([0 1 1 0 0],[0 0 1 1 0]);disp('+');
  poslin : neural/Transfer Functions/poslin
      settings: SourceProductName=Deep Learning Toolbox  SourceProductBaseCode=NN
  "a{1}" : Outport  InitialOutput=[0;0;0;0;0;0;0;0;0;0;0;0;0;0;0;0;0;0;0;0;0;0;0;0;0;0;0;0;0;0;0;0;0;0;0;0;0;0;0;0;0;0;0;0;0;0;0;0;0;0]
      mask Display: e = 0.06;c1 = [120 230 180]/255;c2 = ([120 230 180]+0)/(256+128);c3 = ([120 230 180]+128)/(256+128);patch([0 1 1 0],[0 0 1 1],c1);patch([0 1 1 1-e 1-e 0+e],[0 0 1 1-e 0+e 0+e],c2);patch([0 0 1 1-e 0+e 0+e],[0 1 1 1-e 1-e 0+e],c3);plot([0 1 1 0 0],[0 0 1 1 0]);
  wires:
  poslin/1 -> "a{1}"/1
  netsum/1 -> poslin/1
  "b{1}"/1 -> netsum/2
  "IW{1,1}"/1("iz{1,1}") -> netsum/1
  Delays 1/1("pd{1,1}") -> "IW{1,1}"/1("pd{1,1}")
  "p{1}"/1 -> Delays 1/1("p{1}")

== system AcasXuClosedLoop_all_2017b/networks/Networks_m_5/AcasXu_5_5/Layer 2 ==
  "a{1} " : Inport  PortDimensions=50
      mask Display: e = 0.06;c1 = [120 230 180]/255;c2 = ([120 230 180]+0)/(256+128);c3 = ([120 230 180]+128)/(256+128);patch([0 1 1 0],[0 0 1 1],c1);patch([0 1 1 1-e 1-e 0+e],[0 0 1 1-e 0+e 0+e],c2);patch([0 0 1 1-e 0+e 0+e],[0 1 1 1-e 1-e 0+e],c3);plot([0 1 1 0 0],[0 0 1 1 0]);
  Delays 1 : Subsystem
      mask Display: e = 0.06;c1 = [120 150 230]/255;c2 = ([120 150 230]+0)/383;c3 = ([120 150 230]+128)/383;patch([0 1 1 0],[0 0 1 1],c1);patch([0 1 1 1-e 1-e 0+e],[0 0 1 1-e 0+e 0+e],c2);patch([0 0 1 1-e 0+e 0+e],[0 1 1 1-e 1-e 0+e],c3);plot([0 1 1 0 0],[0 0 1 1 0]);disp('0');
  "LW{2,1}" : Subsystem
      mask Display: e = 0.06;c1 = [120 150 230]/255;c2 = ([120 150 230]+0)/383;c3 = ([120 150 230]+128)/383;patch([0 1 1 0],[0 0 1 1],c1);patch([0 1 1 1-e 1-e 0+e],[0 0 1 1-e 0+e 0+e],c2);patch([0 0 1 1-e 0+e 0+e],[0 1 1 1-e 1-e 0+e],c3);plot([0 1 1 0 0],[0 0 1 1 0]);disp('W');
  "b{2}" : Constant  Value=[0.431130000000000013216094885137863457202911376953125;0.04943609999999999671249639732195646502077579498291015625;0.216195999999999999285904550561099313199520111083984375;-0.75297899999999995390709273124230094254016876220703125;-0.28822700000000001097077984013594686985015869140625;-0.0096169299999999992289989592109122895635664463043212890625;-0.13511200000000000986943859970779158174991607666015625;-0.11357599999999999640376557863419293425977230072021484375;-0.00810956000000000003236078072177406284026801586151123046875;0.116875000000000006661338147750939242541790008544921875;0.335077000000000013724132941206335090100765228271484375;1.2460100000000000619593265582807362079620361328125;-0.03696080000000000198578931076553999446332454681396484375;-0.00818923000000000046727510749633438535965979099273681640625;1.108400000000000051869619710487313568592071533203125;0.210677000000000003154809746774844825267791748046875;-0.280135000000000022879476091475225985050201416015625;-0.00891871999999999977293274611156448372639715671539306640625;-0.028556999999999999051425447760266251862049102783203125;-0.2878089999999999815116780155221931636333465576171875;-0.032944800000000003248334934369268012233078479766845703125;-0.85714100000000004175859658062108792364597320556640625;0.1431179999999999952198237451739259995520114898681640625;0.6268029999999999990478727340814657509326934814453125;-0.446975999999999984435561373175005428493022918701171875;-0.0018736099999999999456135046926874565542675554752349853515625;0.562051999999999996049382389173842966556549072265625;-0.01532219999999999936191041882693752995692193508148193359375;0.646849000000000007304379323613829910755157470703125;0.03280840000000000145075063073818455450236797332763671875;1.507919999999999927098315311013720929622650146484375;-0.4519859999999999988773424774990417063236236572265625;-0.330091999999999996528998735811910592019557952880859375;-1.0895200000000000439825953435502015054225921630859375;0.15831899999999998751576413269503973424434661865234375;0.4723479999999999900950342635042034089565277099609375;0.7157649999999999845812226340058259665966033935546875;-0.62698500000000001453059894629404880106449127197265625;0.1386989999999999889634949568062438629567623138427734375;-0.79259599999999996722266359938657842576503753662109375;-0.79381599999999996608579522217041812837123870849609375;-0.2031389999999999862456689925238606519997119903564453125;0.813736000000000014864554032101295888423919677734375;0.1588219999999999909601200442921253852546215057373046875;-0.002554850000000000002919886554764161701314151287078857421875;0.94193599999999999550226448263856582343578338623046875;-0.252969999999999972661868241630145348608493804931640625;-0.67198999999999997623234548882464878261089324951171875;-0.0283432999999999983564702432659032638184726238250732421875;-0.74789600000000000523669996255193836987018585205078125]
      mask Display: e = 0.06;c1 = [120 150 230]/255;c2 = ([120 150 230]+0)/383;c3 = ([120 150 230]+128)/383;patch([0 1 1 0],[0 0 1 1],c1);patch([0 1 1 1-e 1-e 0+e],[0 0 1 1-e 0+e 0+e],c2);patch([0 0 1 1-e 0+e 0+e],[0 1 1 1-e 1-e 0+e],c3);plot([0 1 1 0 0],[0 0 1 1 0]);disp('b');
  netsum : Sum  Inputs=2  SaturateOnIntegerOverflow=on  InputSameDT=on  OutDataTypeStr=Inherit: Same as first input
      mask Display: e = 0.06;c1 = [120 150 230]/255;c2 = ([120 150 230]+0)/383;c3 = ([120 150 230]+128)/383;patch([0 1 1 0],[0 0 1 1],c1);patch([0 1 1 1-e 1-e 0+e],[0 0 1 1-e 0+e 0+e],c2);patch([0 0 1 1-e 0+e 0+e],[0 1 1 1-e 1-e 0+e],c3);plot([0 1 1 0 0],[0 0 1 1 0]);disp('+');
  poslin : neural/Transfer Functions/poslin
      settings: SourceProductName=Deep Learning Toolbox  SourceProductBaseCode=NN
  "a{2}" : Outport  InitialOutput=[0;0;0;0;0;0;0;0;0;0;0;0;0;0;0;0;0;0;0;0;0;0;0;0;0;0;0;0;0;0;0;0;0;0;0;0;0;0;0;0;0;0;0;0;0;0;0;0;0;0]
      mask Display: e = 0.06;c1 = [120 230 180]/255;c2 = ([120 230 180]+0)/(256+128);c3 = ([120 230 180]+128)/(256+128);patch([0 1 1 0],[0 0 1 1],c1);patch([0 1 1 1-e 1-e 0+e],[0 0 1 1-e 0+e 0+e],c2);patch([0 0 1 1-e 0+e 0+e],[0 1 1 1-e 1-e 0+e],c3);plot([0 1 1 0 0],[0 0 1 1 0]);
  wires:
  poslin/1 -> "a{2}"/1
  netsum/1 -> poslin/1
  "b{2}"/1 -> netsum/2
  "LW{2,1}"/1("lz{2,1}") -> netsum/1
  Delays 1/1("pd{2,1}") -> "LW{2,1}"/1("ad{2,1}")
  "a{1} "/1 -> Delays 1/1("p{2}")
